simulink model "ActvnCoorrForEcuAndCom"  (R2022b)
settings: Solver=FixedStepDiscrete  StartTime=0.0  StopTime=10.0  FixedStep=0.1  RelTol=1e-3
settings Model: Creator=blue.ge  LastModifiedBy=blue.ge
settings DataIOCC: SaveFormat=StructureWithTime
settings OptimizationCC: DefaultParameterBehavior=Inlined  EnhancedBackFolding=on  PassReuseOutputArgsAs=Individual arguments  ZeroExternalMemoryAtStartup=off  ZeroInternalMemoryAtStartup=off  OptimizeBlockOrder=speed  DifferentSizesBufferReuse=on  OptimizationCustomize=off  EfficientTunableParamExpr=on
settings DebuggingCC: ParameterTunabilityLossMsg=error
settings ModelReferenceCC: ModelReferenceNumInstancesAllowed=Single
settings SFSimCC: MATLABDynamicMemAlloc=off
settings RTWCC: Description=AUTOSAR  SystemTargetFile=autosar.tlc  GenCodeOnly=on  TemplateMakefile=ert_default_tmf
settings CodeAppCC: ForceParamTrailComments=on  IgnoreCustomStorageClasses=off  OperatorAnnotations=on  SimulinkDataObjDesc=on  SFDataObjDesc=on  InsertBlockDesc=on
settings STFCustomTargetCC: TargetLangStandard=C99 (ISO)  MultiwordLength=256  ParMdlRefBuildCompliant=on  CompOptLevelCompliant=on  IncludeMdlTerminateFcn=off  CombineOutputUpdateFcns=on  SuppressErrorStatus=on  ERTCustomFileBanners=on  SupportNonFinite=off  SupportComplex=off  SupportContinuousTime=off  SupportNonInlinedSFcns=off  RemoveResetFunc=on  MATLABClassNameForMDSCustomization=Simulink.SoftwareTarget.ERTCustomization  AutosarCompliant=on  UseToolchainInfoCompliant=on  LookupTableObjectStructAxisOrder=2,1,3,4,...  LUTObjectStructOrderEvenSpacing=Size,Table,Breakpoints  SystemTargetFile=autosar.tlc
settings ERTTargetCC: TargetLangStandard=C99 (ISO)  ConcurrentExecutionCompliant=off  IncludeMdlTerminateFcn=off  SuppressErrorStatus=on  SupportNonFinite=off  SupportComplex=off  ModelStepFunctionPrototypeControlCompliant=off  CPPClassGenCompliant=off  AutosarCompliant=on  LookupTableObjectStructAxisOrder=2,1,3,4,...  LUTObjectStructOrderEvenSpacing=Size,Table,Breakpoints  InlinedParameterPlacement=NonHierarchical  ERTCustomFileTemplate=example_file_process.tlc

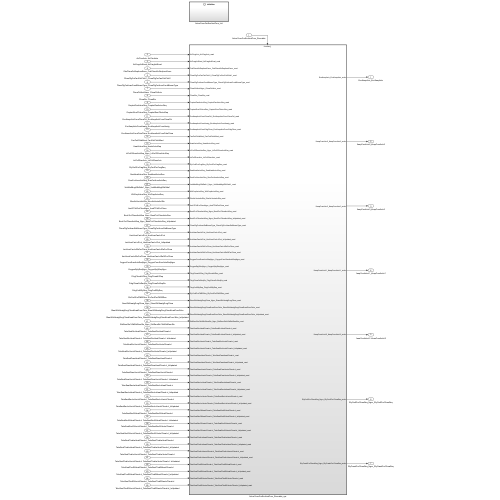
[thumbnail: Simulink's stored preview of the top-level diagram]
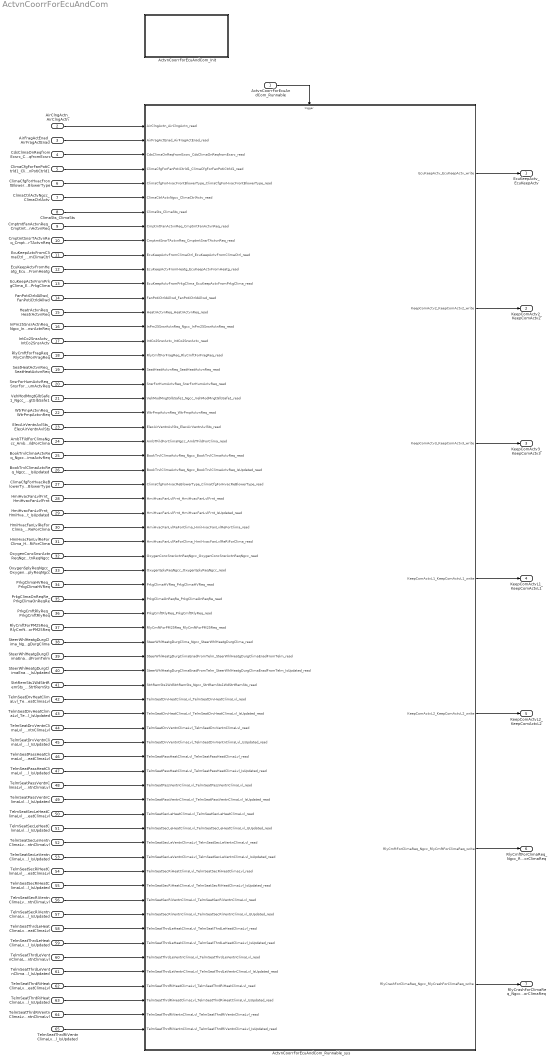
== system ActvnCoorrForEcuAndCom ==
  ActvnCoorrForEcuAndCom_Runnable : Inport  SampleTime=0.1  OutputFunctionCall=on
  AirClngActn_AirClngActn : Inport  Port=2  OutDataTypeStr=Enum: OnOff1  PortDimensions=1
  AirFragActEnad_AirFragActEnad : Inport  Port=3  OutDataTypeStr=Enum: OnOff1  PortDimensions=1
  CdsClimaOnReqfromExsrc_CdsClimaOnReqfromExsrc : Inport  Port=4  OutDataTypeStr=Enum: OnOff1  PortDimensions=1
  ClimaCfgForFanPotiCtrld1_ClimaCfgForFanPotiCtrld1 : Inport  Port=5  OutDataTypeStr=Boolean  PortDimensions=1
  ClimaCfgForHvacFrontBlowerType_ClimaCfgForHvacFrontBlowerType : Inport  Port=6  OutDataTypeStr=Enum: ClimaCfgForHvacFrontBlowerType  PortDimensions=1
  ClimaCtrlActvNgcc_ClimaCtrlActv : Inport  Port=7  OutDataTypeStr=Boolean  PortDimensions=1
  ClimaSts_ClimaSts : Inport  Port=8  OutDataTypeStr=Enum: ClimaSts  PortDimensions=1
  CmptmtFanActvnReq_CmptmtFanActvnReq : Inport  Port=9  OutDataTypeStr=Enum: OnOff1  PortDimensions=1
  CmptmtSnsrTActvnReq_CmptmtSnsrTActvnReq : Inport  Port=10  OutDataTypeStr=Enum: OnOff1  PortDimensions=1
  EcuKeepActvFromClimaCtrl_EcuKeepActvFromClimaCtrl : Inport  Port=11  OutDataTypeStr=Enum: OnOff1  PortDimensions=1
  EcuKeepActvFromHeatg_EcuKeepActvFromHeatg : Inport  Port=12  OutDataTypeStr=Enum: OnOff1  PortDimensions=1
  EcuKeepActvFromPrkgClima_EcuKeepActvFromPrkgClima : Inport  Port=13  OutDataTypeStr=Boolean  PortDimensions=1
  FanPotiCtrldAllwd_FanPotiCtrldAllwd : Inport  Port=14  OutDataTypeStr=Boolean  PortDimensions=1
  HeatrActvnReq_HeatrActvnReq : Inport  Port=15  OutDataTypeStr=Enum: OnOff1  PortDimensions=1
  InPm25SnsrActnReq_Ngcc_InPm25SnsrActnReq : Inport  Port=16  OutDataTypeStr=Enum: OnOff1  PortDimensions=1
  IntCo2SnsrActv_IntCo2SnsrActv : Inport  Port=17  OutDataTypeStr=Enum: ReqSts1  PortDimensions=1
  RlyCmftForFragReq_RlyCmftForFragReq : Inport  Port=18  OutDataTypeStr=Enum: OnOff1  PortDimensions=1
  SeatHeatActvnReq_SeatHeatActvnReq : Inport  Port=19  OutDataTypeStr=Enum: OnOff1  PortDimensions=1
  SnsrForHumActvReq_SnsrForHumActvReq : Inport  Port=20  OutDataTypeStr=Enum: OnOff1  PortDimensions=1
  VehModMngtGlbSafe1_Ngcc_VehModMngtGlbSafe1 : Inport  Port=21  OutDataTypeStr=Bus: VehModMngtGlbSafe1  PortDimensions=1  BusOutputAsStruct=on
  WtrPmpActvnReq_WtrPmpActvnReq : Inport  Port=22  OutDataTypeStr=Enum: OnOff1  PortDimensions=1
  ElecAirVentnAvlSts_ElecAirVentnAvlSts : Inport  Port=23  OutDataTypeStr=Enum: StsFd  PortDimensions=1
  AmbTFildForClimaNgcc_AmbTFildForClima : Inport  Port=24  OutDataTypeStr=T30  PortDimensions=1
  BookTrvlClimaActvReq_Ngcc_BookTrvlClimaActvReq : Inport  Port=25  OutDataTypeStr=Enum: OnOffNoReq  PortDimensions=1
  BookTrvlClimaActvReq_Ngcc_BookTrvlClimaActvReq_IsUpdated : Inport  Port=26  OutDataTypeStr=boolean  PortDimensions=1
  ClimaCfgForHvacReBlowerType_ClimaCfgForHvacReBlowerType : Inport  Port=27  OutDataTypeStr=Enum: ClimaCfgForHvacReBlowerType  PortDimensions=1
  HmiHvacFanLvlFrnt_HmiHvacFanLvlFrnt : Inport  Port=28  OutDataTypeStr=Enum: HmiHvacFanLvl  PortDimensions=1
  HmiHvacFanLvlFrnt_HmiHvacFanLvlFrnt_IsUpdated : Inport  Port=29  OutDataTypeStr=boolean  PortDimensions=1
  HmiHvacFanLvlReForClima_HmiHvacFanLvlReForClima : Inport  Port=30  OutDataTypeStr=Enum: HmiHvacFanLvl  PortDimensions=1
  HmiHvacFanLvlReForClima_HmiHvacFanLvlReRiForClima : Inport  Port=31  OutDataTypeStr=Enum: HmiHvacFanLvl  PortDimensions=1
  OxygenConcSnsrActnReqNgcc_OxygenConcSnsrActnReqNgcc : Inport  Port=32  OutDataTypeStr=Enum: OnOff1  PortDimensions=1
  OxygenSplyReqNgcc_OxygenSplyReqNgcc : Inport  Port=33  OutDataTypeStr=Enum: OxygenSplyReq  PortDimensions=1
  PrkgClimaHVReq_PrkgClimaHVReq : Inport  Port=34  OutDataTypeStr=Enum: OnOff1  PortDimensions=1
  PrkgClimaOnReqRe_PrkgClimaOnReqRe : Inport  Port=35  OutDataTypeStr=Enum: OnOff1  PortDimensions=1
  PrkgCmftRlyReq_PrkgCmftRlyReq : Inport  Port=36  OutDataTypeStr=Boolean  PortDimensions=1
  RlyCmftForPM25Req_RlyCmftForPM25Req : Inport  Port=37  OutDataTypeStr=Enum: OnOff1  PortDimensions=1
  SteerWhlHeatgDurgClima_Ngcc_SteerWhlHeatgDurgClima : Inport  Port=38  OutDataTypeStr=Boolean  PortDimensions=1
  SteerWhlHeatgDurgClimaEnadFromTelm_SteerWhlHeatgDurgClimaEnadFromTelm : Inport  Port=39  OutDataTypeStr=Enum: OnOff1  PortDimensions=1
  SteerWhlHeatgDurgClimaEnadFromTelm_SteerWhlHeatgDurgClimaEnadFromTelm_IsUpdated : Inport  Port=40  OutDataTypeStr=boolean  PortDimensions=1
  StrtRemSts1WdStrtRemSts_Ngcc_StrtRemSts1WdStrtRemSts : Inport  Port=41  OutDataTypeStr=Enum: StrtRemSts1  PortDimensions=1
  TelmSeatDrvHeatClimaLvl_TelmSeatDrvHeatClimaLvl : Inport  Port=42  OutDataTypeStr=Enum: TelmSeatClimaLvl  PortDimensions=1
  TelmSeatDrvHeatClimaLvl_TelmSeatDrvHeatClimaLvl_IsUpdated : Inport  Port=43  OutDataTypeStr=boolean  PortDimensions=1
  TelmSeatDrvVentnClimaLvl_TelmSeatDrvVentnClimaLvl : Inport  Port=44  OutDataTypeStr=Enum: TelmSeatClimaLvl  PortDimensions=1
  TelmSeatDrvVentnClimaLvl_TelmSeatDrvVentnClimaLvl_IsUpdated : Inport  Port=45  OutDataTypeStr=boolean  PortDimensions=1
  TelmSeatPassHeatClimaLvl_TelmSeatPassHeatClimaLvl : Inport  Port=46  OutDataTypeStr=Enum: TelmSeatClimaLvl  PortDimensions=1
  TelmSeatPassHeatClimaLvl_TelmSeatPassHeatClimaLvl_IsUpdated : Inport  Port=47  OutDataTypeStr=boolean  PortDimensions=1
  TelmSeatPassVentnClimaLvl_TelmSeatPassVentnClimaLvl : Inport  Port=48  OutDataTypeStr=Enum: TelmSeatClimaLvl  PortDimensions=1
  TelmSeatPassVentnClimaLvl_TelmSeatPassVentnClimaLvl_IsUpdated : Inport  Port=49  OutDataTypeStr=boolean  PortDimensions=1
  TelmSeatSecLeHeatClimaLvl_TelmSeatSecLeHeatClimaLvl : Inport  Port=50  OutDataTypeStr=Enum: TelmSeatClimaLvl  PortDimensions=1
  TelmSeatSecLeHeatClimaLvl_TelmSeatSecLeHeatClimaLvl_IsUpdated : Inport  Port=51  OutDataTypeStr=boolean  PortDimensions=1
  TelmSeatSecLeVentnClimaLvl_TelmSeatSecLeVentnClimaLvl : Inport  Port=52  OutDataTypeStr=Enum: TelmSeatClimaLvl  PortDimensions=1
  TelmSeatSecLeVentnClimaLvl_TelmSeatSecLeVentnClimaLvl_IsUpdated : Inport  Port=53  OutDataTypeStr=boolean  PortDimensions=1
  TelmSeatSecRiHeatClimaLvl_TelmSeatSecRiHeatClimaLvl : Inport  Port=54  OutDataTypeStr=Enum: TelmSeatClimaLvl  PortDimensions=1
  TelmSeatSecRiHeatClimaLvl_TelmSeatSecRiHeatClimaLvl_IsUpdated : Inport  Port=55  OutDataTypeStr=boolean  PortDimensions=1
  TelmSeatSecRiVentnClimaLvl_TelmSeatSecRiVentnClimaLvl : Inport  Port=56  OutDataTypeStr=Enum: TelmSeatClimaLvl  PortDimensions=1
  TelmSeatSecRiVentnClimaLvl_TelmSeatSecRiVentnClimaLvl_IsUpdated : Inport  Port=57  OutDataTypeStr=boolean  PortDimensions=1
  TelmSeatThrdLeHeatClimaLvl_TelmSeatThrdLeHeatClimaLvl : Inport  Port=58  OutDataTypeStr=Enum: TelmSeatClimaLvl  PortDimensions=1
  TelmSeatThrdLeHeatClimaLvl_TelmSeatThrdLeHeatClimaLvl_IsUpdated : Inport  Port=59  OutDataTypeStr=boolean  PortDimensions=1
  TelmSeatThrdLeVentnClimaLvl_TelmSeatThrdLeVentnClimaLvl : Inport  Port=60  OutDataTypeStr=Enum: TelmSeatClimaLvl  PortDimensions=1
  TelmSeatThrdLeVentnClimaLvl_TelmSeatThrdLeVentnClimaLvl_IsUpdated : Inport  Port=61  OutDataTypeStr=boolean  PortDimensions=1
  TelmSeatThrdRiHeatClimaLvl_TelmSeatThrdRiHeatClimaLvl : Inport  Port=62  OutDataTypeStr=Enum: TelmSeatClimaLvl  PortDimensions=1
  TelmSeatThrdRiHeatClimaLvl_TelmSeatThrdRiHeatClimaLvl_IsUpdated : Inport  Port=63  OutDataTypeStr=boolean  PortDimensions=1
  TelmSeatThrdRiVentnClimaLvl_TelmSeatThrdRiVentnClimaLvl : Inport  Port=64  OutDataTypeStr=Enum: TelmSeatClimaLvl  PortDimensions=1
  TelmSeatThrdRiVentnClimaLvl_TelmSeatThrdRiVentnClimaLvl_IsUpdated : Inport  Port=65  OutDataTypeStr=boolean  PortDimensions=1
  ActvnCoorrForEcuAndCom_Init : Subsystem
  ActvnCoorrForEcuAndCom_Runnable_sys : Subsystem
  EcuKeepActv_EcuKeepActv : Outport  OutDataTypeStr=Enum: OnOff1  PortDimensions=1
  KeepComActv2_KeepComActv2 : Outport  Port=2  OutDataTypeStr=Enum: OnOff1  PortDimensions=1
  KeepComActv3_KeepComActv3 : Outport  Port=3  OutDataTypeStr=Enum: OnOff1  PortDimensions=1
  KeepComActvL1_KeepComActvL1 : Outport  Port=4  OutDataTypeStr=Enum: OnOff1  PortDimensions=1
  KeepComActvL2_KeepComActvL2 : Outport  Port=5  OutDataTypeStr=Enum: OnOff1  PortDimensions=1
  RlyCmftForClimaReq_Ngcc_RlyCmftForClimaReq : Outport  Port=6  OutDataTypeStr=Enum: OnOff1  PortDimensions=1
  RlyCrashForClimaReq_Ngcc_RlyCrashForClimaReq : Outport  Port=7  OutDataTypeStr=Enum: OnOff1  PortDimensions=1
  wires:
  AirClngActn_AirClngActn/1 -> ActvnCoorrForEcuAndCom_Runnable_sys/1(AirClngActn_AirClngActn_read)
  AirFragActEnad_AirFragActEnad/1 -> ActvnCoorrForEcuAndCom_Runnable_sys/2(AirFragActEnad_AirFragActEnad_read)
  CdsClimaOnReqfromExsrc_CdsClimaOnReqfromExsrc/1 -> ActvnCoorrForEcuAndCom_Runnable_sys/3(CdsClimaOnReqfromExsrc_CdsClimaOnReqfromExsrc_read)
  ClimaCfgForFanPotiCtrld1_ClimaCfgForFanPotiCtrld1/1 -> ActvnCoorrForEcuAndCom_Runnable_sys/4(ClimaCfgForFanPotiCtrld1_ClimaCfgForFanPotiCtrld1_read)
  ClimaCfgForHvacFrontBlowerType_ClimaCfgForHvacFrontBlowerType/1 -> ActvnCoorrForEcuAndCom_Runnable_sys/5(ClimaCfgForHvacFrontBlowerType_ClimaCfgForHvacFrontBlowerType_read)
  ClimaCtrlActvNgcc_ClimaCtrlActv/1 -> ActvnCoorrForEcuAndCom_Runnable_sys/6(ClimaCtrlActvNgcc_ClimaCtrlActv_read)
  ClimaSts_ClimaSts/1 -> ActvnCoorrForEcuAndCom_Runnable_sys/7(ClimaSts_ClimaSts_read)
  CmptmtFanActvnReq_CmptmtFanActvnReq/1 -> ActvnCoorrForEcuAndCom_Runnable_sys/8(CmptmtFanActvnReq_CmptmtFanActvnReq_read)
  CmptmtSnsrTActvnReq_CmptmtSnsrTActvnReq/1 -> ActvnCoorrForEcuAndCom_Runnable_sys/9(CmptmtSnsrTActvnReq_CmptmtSnsrTActvnReq_read)
  EcuKeepActvFromClimaCtrl_EcuKeepActvFromClimaCtrl/1 -> ActvnCoorrForEcuAndCom_Runnable_sys/10(EcuKeepActvFromClimaCtrl_EcuKeepActvFromClimaCtrl_read)
  EcuKeepActvFromHeatg_EcuKeepActvFromHeatg/1 -> ActvnCoorrForEcuAndCom_Runnable_sys/11(EcuKeepActvFromHeatg_EcuKeepActvFromHeatg_read)
  EcuKeepActvFromPrkgClima_EcuKeepActvFromPrkgClima/1 -> ActvnCoorrForEcuAndCom_Runnable_sys/12(EcuKeepActvFromPrkgClima_EcuKeepActvFromPrkgClima_read)
  FanPotiCtrldAllwd_FanPotiCtrldAllwd/1 -> ActvnCoorrForEcuAndCom_Runnable_sys/13(FanPotiCtrldAllwd_FanPotiCtrldAllwd_read)
  HeatrActvnReq_HeatrActvnReq/1 -> ActvnCoorrForEcuAndCom_Runnable_sys/14(HeatrActvnReq_HeatrActvnReq_read)
  InPm25SnsrActnReq_Ngcc_InPm25SnsrActnReq/1 -> ActvnCoorrForEcuAndCom_Runnable_sys/15(InPm25SnsrActnReq_Ngcc_InPm25SnsrActnReq_read)
  IntCo2SnsrActv_IntCo2SnsrActv/1 -> ActvnCoorrForEcuAndCom_Runnable_sys/16(IntCo2SnsrActv_IntCo2SnsrActv_read)
  RlyCmftForFragReq_RlyCmftForFragReq/1 -> ActvnCoorrForEcuAndCom_Runnable_sys/17(RlyCmftForFragReq_RlyCmftForFragReq_read)
  SeatHeatActvnReq_SeatHeatActvnReq/1 -> ActvnCoorrForEcuAndCom_Runnable_sys/18(SeatHeatActvnReq_SeatHeatActvnReq_read)
  SnsrForHumActvReq_SnsrForHumActvReq/1 -> ActvnCoorrForEcuAndCom_Runnable_sys/19(SnsrForHumActvReq_SnsrForHumActvReq_read)
  VehModMngtGlbSafe1_Ngcc_VehModMngtGlbSafe1/1 -> ActvnCoorrForEcuAndCom_Runnable_sys/20(VehModMngtGlbSafe1_Ngcc_VehModMngtGlbSafe1_read)
  WtrPmpActvnReq_WtrPmpActvnReq/1 -> ActvnCoorrForEcuAndCom_Runnable_sys/21(WtrPmpActvnReq_WtrPmpActvnReq_read)
  ElecAirVentnAvlSts_ElecAirVentnAvlSts/1 -> ActvnCoorrForEcuAndCom_Runnable_sys/22(ElecAirVentnAvlSts_ElecAirVentnAvlSts_read)
  AmbTFildForClimaNgcc_AmbTFildForClima/1 -> ActvnCoorrForEcuAndCom_Runnable_sys/23(AmbTFildForClimaNgcc_AmbTFildForClima_read)
  BookTrvlClimaActvReq_Ngcc_BookTrvlClimaActvReq/1 -> ActvnCoorrForEcuAndCom_Runnable_sys/24(BookTrvlClimaActvReq_Ngcc_BookTrvlClimaActvReq_read)
  BookTrvlClimaActvReq_Ngcc_BookTrvlClimaActvReq_IsUpdated/1 -> ActvnCoorrForEcuAndCom_Runnable_sys/25(BookTrvlClimaActvReq_Ngcc_BookTrvlClimaActvReq_IsUpdated_read)
  ClimaCfgForHvacReBlowerType_ClimaCfgForHvacReBlowerType/1 -> ActvnCoorrForEcuAndCom_Runnable_sys/26(ClimaCfgForHvacReBlowerType_ClimaCfgForHvacReBlowerType_read)
  HmiHvacFanLvlFrnt_HmiHvacFanLvlFrnt/1 -> ActvnCoorrForEcuAndCom_Runnable_sys/27(HmiHvacFanLvlFrnt_HmiHvacFanLvlFrnt_read)
  HmiHvacFanLvlFrnt_HmiHvacFanLvlFrnt_IsUpdated/1 -> ActvnCoorrForEcuAndCom_Runnable_sys/28(HmiHvacFanLvlFrnt_HmiHvacFanLvlFrnt_IsUpdated_read)
  HmiHvacFanLvlReForClima_HmiHvacFanLvlReForClima/1 -> ActvnCoorrForEcuAndCom_Runnable_sys/29(HmiHvacFanLvlReForClima_HmiHvacFanLvlReForClima_read)
  HmiHvacFanLvlReForClima_HmiHvacFanLvlReRiForClima/1 -> ActvnCoorrForEcuAndCom_Runnable_sys/30(HmiHvacFanLvlReForClima_HmiHvacFanLvlReRiForClima_read)
  OxygenConcSnsrActnReqNgcc_OxygenConcSnsrActnReqNgcc/1 -> ActvnCoorrForEcuAndCom_Runnable_sys/31(OxygenConcSnsrActnReqNgcc_OxygenConcSnsrActnReqNgcc_read)
  OxygenSplyReqNgcc_OxygenSplyReqNgcc/1 -> ActvnCoorrForEcuAndCom_Runnable_sys/32(OxygenSplyReqNgcc_OxygenSplyReqNgcc_read)
  PrkgClimaHVReq_PrkgClimaHVReq/1 -> ActvnCoorrForEcuAndCom_Runnable_sys/33(PrkgClimaHVReq_PrkgClimaHVReq_read)
  PrkgClimaOnReqRe_PrkgClimaOnReqRe/1 -> ActvnCoorrForEcuAndCom_Runnable_sys/34(PrkgClimaOnReqRe_PrkgClimaOnReqRe_read)
  PrkgCmftRlyReq_PrkgCmftRlyReq/1 -> ActvnCoorrForEcuAndCom_Runnable_sys/35(PrkgCmftRlyReq_PrkgCmftRlyReq_read)
  RlyCmftForPM25Req_RlyCmftForPM25Req/1 -> ActvnCoorrForEcuAndCom_Runnable_sys/36(RlyCmftForPM25Req_RlyCmftForPM25Req_read)
  SteerWhlHeatgDurgClima_Ngcc_SteerWhlHeatgDurgClima/1 -> ActvnCoorrForEcuAndCom_Runnable_sys/37(SteerWhlHeatgDurgClima_Ngcc_SteerWhlHeatgDurgClima_read)
  SteerWhlHeatgDurgClimaEnadFromTelm_SteerWhlHeatgDurgClimaEnadFromTelm/1 -> ActvnCoorrForEcuAndCom_Runnable_sys/38(SteerWhlHeatgDurgClimaEnadFromTelm_SteerWhlHeatgDurgClimaEnadFromTelm_read)
  SteerWhlHeatgDurgClimaEnadFromTelm_SteerWhlHeatgDurgClimaEnadFromTelm_IsUpdated/1 -> ActvnCoorrForEcuAndCom_Runnable_sys/39(SteerWhlHeatgDurgClimaEnadFromTelm_SteerWhlHeatgDurgClimaEnadFromTelm_IsUpdated_read)
  StrtRemSts1WdStrtRemSts_Ngcc_StrtRemSts1WdStrtRemSts/1 -> ActvnCoorrForEcuAndCom_Runnable_sys/40(StrtRemSts1WdStrtRemSts_Ngcc_StrtRemSts1WdStrtRemSts_read)
  TelmSeatDrvHeatClimaLvl_TelmSeatDrvHeatClimaLvl/1 -> ActvnCoorrForEcuAndCom_Runnable_sys/41(TelmSeatDrvHeatClimaLvl_TelmSeatDrvHeatClimaLvl_read)
  TelmSeatDrvHeatClimaLvl_TelmSeatDrvHeatClimaLvl_IsUpdated/1 -> ActvnCoorrForEcuAndCom_Runnable_sys/42(TelmSeatDrvHeatClimaLvl_TelmSeatDrvHeatClimaLvl_IsUpdated_read)
  TelmSeatDrvVentnClimaLvl_TelmSeatDrvVentnClimaLvl/1 -> ActvnCoorrForEcuAndCom_Runnable_sys/43(TelmSeatDrvVentnClimaLvl_TelmSeatDrvVentnClimaLvl_read)
  TelmSeatDrvVentnClimaLvl_TelmSeatDrvVentnClimaLvl_IsUpdated/1 -> ActvnCoorrForEcuAndCom_Runnable_sys/44(TelmSeatDrvVentnClimaLvl_TelmSeatDrvVentnClimaLvl_IsUpdated_read)
  TelmSeatPassHeatClimaLvl_TelmSeatPassHeatClimaLvl/1 -> ActvnCoorrForEcuAndCom_Runnable_sys/45(TelmSeatPassHeatClimaLvl_TelmSeatPassHeatClimaLvl_read)
  TelmSeatPassHeatClimaLvl_TelmSeatPassHeatClimaLvl_IsUpdated/1 -> ActvnCoorrForEcuAndCom_Runnable_sys/46(TelmSeatPassHeatClimaLvl_TelmSeatPassHeatClimaLvl_IsUpdated_read)
  TelmSeatPassVentnClimaLvl_TelmSeatPassVentnClimaLvl/1 -> ActvnCoorrForEcuAndCom_Runnable_sys/47(TelmSeatPassVentnClimaLvl_TelmSeatPassVentnClimaLvl_read)
  TelmSeatPassVentnClimaLvl_TelmSeatPassVentnClimaLvl_IsUpdated/1 -> ActvnCoorrForEcuAndCom_Runnable_sys/48(TelmSeatPassVentnClimaLvl_TelmSeatPassVentnClimaLvl_IsUpdated_read)
  TelmSeatSecLeHeatClimaLvl_TelmSeatSecLeHeatClimaLvl/1 -> ActvnCoorrForEcuAndCom_Runnable_sys/49(TelmSeatSecLeHeatClimaLvl_TelmSeatSecLeHeatClimaLvl_read)
  TelmSeatSecLeHeatClimaLvl_TelmSeatSecLeHeatClimaLvl_IsUpdated/1 -> ActvnCoorrForEcuAndCom_Runnable_sys/50(TelmSeatSecLeHeatClimaLvl_TelmSeatSecLeHeatClimaLvl_IsUpdated_read)
  TelmSeatSecLeVentnClimaLvl_TelmSeatSecLeVentnClimaLvl/1 -> ActvnCoorrForEcuAndCom_Runnable_sys/51(TelmSeatSecLeVentnClimaLvl_TelmSeatSecLeVentnClimaLvl_read)
  TelmSeatSecLeVentnClimaLvl_TelmSeatSecLeVentnClimaLvl_IsUpdated/1 -> ActvnCoorrForEcuAndCom_Runnable_sys/52(TelmSeatSecLeVentnClimaLvl_TelmSeatSecLeVentnClimaLvl_IsUpdated_read)
  TelmSeatSecRiHeatClimaLvl_TelmSeatSecRiHeatClimaLvl/1 -> ActvnCoorrForEcuAndCom_Runnable_sys/53(TelmSeatSecRiHeatClimaLvl_TelmSeatSecRiHeatClimaLvl_read)
  TelmSeatSecRiHeatClimaLvl_TelmSeatSecRiHeatClimaLvl_IsUpdated/1 -> ActvnCoorrForEcuAndCom_Runnable_sys/54(TelmSeatSecRiHeatClimaLvl_TelmSeatSecRiHeatClimaLvl_IsUpdated_read)
  TelmSeatSecRiVentnClimaLvl_TelmSeatSecRiVentnClimaLvl/1 -> ActvnCoorrForEcuAndCom_Runnable_sys/55(TelmSeatSecRiVentnClimaLvl_TelmSeatSecRiVentnClimaLvl_read)
  TelmSeatSecRiVentnClimaLvl_TelmSeatSecRiVentnClimaLvl_IsUpdated/1 -> ActvnCoorrForEcuAndCom_Runnable_sys/56(TelmSeatSecRiVentnClimaLvl_TelmSeatSecRiVentnClimaLvl_IsUpdated_read)
  TelmSeatThrdLeHeatClimaLvl_TelmSeatThrdLeHeatClimaLvl/1 -> ActvnCoorrForEcuAndCom_Runnable_sys/57(TelmSeatThrdLeHeatClimaLvl_TelmSeatThrdLeHeatClimaLvl_read)
  TelmSeatThrdLeHeatClimaLvl_TelmSeatThrdLeHeatClimaLvl_IsUpdated/1 -> ActvnCoorrForEcuAndCom_Runnable_sys/58(TelmSeatThrdLeHeatClimaLvl_TelmSeatThrdLeHeatClimaLvl_IsUpdated_read)
  TelmSeatThrdLeVentnClimaLvl_TelmSeatThrdLeVentnClimaLvl/1 -> ActvnCoorrForEcuAndCom_Runnable_sys/59(TelmSeatThrdLeVentnClimaLvl_TelmSeatThrdLeVentnClimaLvl_read)
  TelmSeatThrdLeVentnClimaLvl_TelmSeatThrdLeVentnClimaLvl_IsUpdated/1 -> ActvnCoorrForEcuAndCom_Runnable_sys/60(TelmSeatThrdLeVentnClimaLvl_TelmSeatThrdLeVentnClimaLvl_IsUpdated_read)
  TelmSeatThrdRiHeatClimaLvl_TelmSeatThrdRiHeatClimaLvl/1 -> ActvnCoorrForEcuAndCom_Runnable_sys/61(TelmSeatThrdRiHeatClimaLvl_TelmSeatThrdRiHeatClimaLvl_read)
  TelmSeatThrdRiHeatClimaLvl_TelmSeatThrdRiHeatClimaLvl_IsUpdated/1 -> ActvnCoorrForEcuAndCom_Runnable_sys/62(TelmSeatThrdRiHeatClimaLvl_TelmSeatThrdRiHeatClimaLvl_IsUpdated_read)
  TelmSeatThrdRiVentnClimaLvl_TelmSeatThrdRiVentnClimaLvl/1 -> ActvnCoorrForEcuAndCom_Runnable_sys/63(TelmSeatThrdRiVentnClimaLvl_TelmSeatThrdRiVentnClimaLvl_read)
  TelmSeatThrdRiVentnClimaLvl_TelmSeatThrdRiVentnClimaLvl_IsUpdated/1 -> ActvnCoorrForEcuAndCom_Runnable_sys/64(TelmSeatThrdRiVentnClimaLvl_TelmSeatThrdRiVentnClimaLvl_IsUpdated_read)
  ActvnCoorrForEcuAndCom_Runnable_sys/1(EcuKeepActv_EcuKeepActv_write) -> EcuKeepActv_EcuKeepActv/1
  ActvnCoorrForEcuAndCom_Runnable_sys/2(KeepComActv2_KeepComActv2_write) -> KeepComActv2_KeepComActv2/1
  ActvnCoorrForEcuAndCom_Runnable_sys/3(KeepComActv3_KeepComActv3_write) -> KeepComActv3_KeepComActv3/1
  ActvnCoorrForEcuAndCom_Runnable_sys/4(KeepComActvL1_KeepComActvL1_write) -> KeepComActvL1_KeepComActvL1/1
  ActvnCoorrForEcuAndCom_Runnable_sys/5(KeepComActvL2_KeepComActvL2_write) -> KeepComActvL2_KeepComActvL2/1
  ActvnCoorrForEcuAndCom_Runnable_sys/6(RlyCmftForClimaReq_Ngcc_RlyCmftForClimaReq_write) -> RlyCmftForClimaReq_Ngcc_RlyCmftForClimaReq/1
  ActvnCoorrForEcuAndCom_Runnable_sys/7(RlyCrashForClimaReq_Ngcc_RlyCrashForClimaReq_write) -> RlyCrashForClimaReq_Ngcc_RlyCrashForClimaReq/1
  ActvnCoorrForEcuAndCom_Runnable/1 -> ActvnCoorrForEcuAndCom_Runnable_sys/Trigger

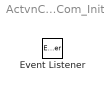
== system ActvnCoorrForEcuAndCom/ActvnCoorrForEcuAndCom_Init ==
  Event Listener : EventListener  EventName=reset

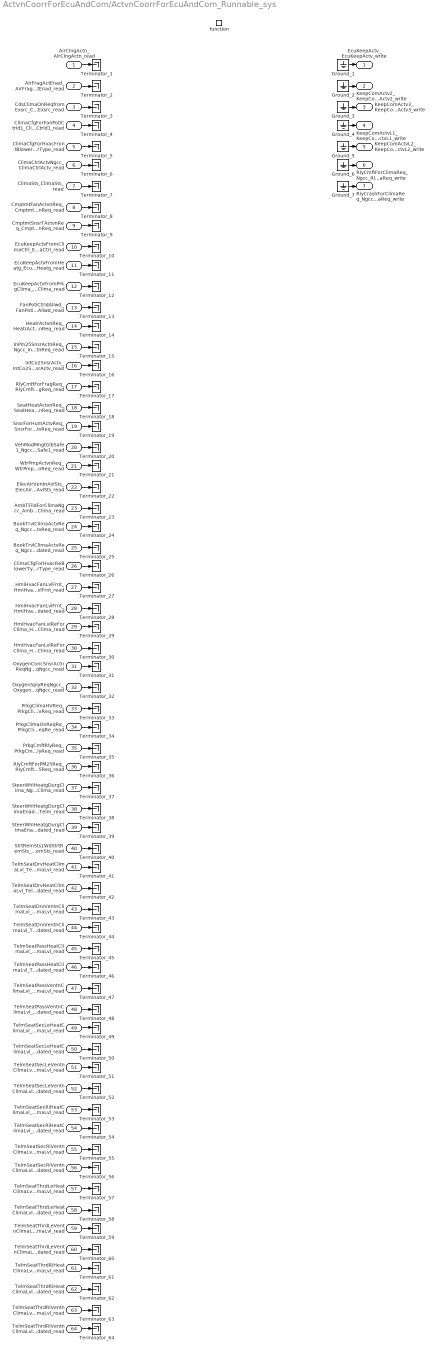
== system ActvnCoorrForEcuAndCom/ActvnCoorrForEcuAndCom_Runnable_sys ==
  AirClngActn_AirClngActn_read : Inport
  AirFragActEnad_AirFragActEnad_read : Inport  Port=2
  CdsClimaOnReqfromExsrc_CdsClimaOnReqfromExsrc_read : Inport  Port=3
  ClimaCfgForFanPotiCtrld1_ClimaCfgForFanPotiCtrld1_read : Inport  Port=4
  ClimaCfgForHvacFrontBlowerType_ClimaCfgForHvacFrontBlowerType_read : Inport  Port=5
  ClimaCtrlActvNgcc_ClimaCtrlActv_read : Inport  Port=6
  ClimaSts_ClimaSts_read : Inport  Port=7
  CmptmtFanActvnReq_CmptmtFanActvnReq_read : Inport  Port=8
  CmptmtSnsrTActvnReq_CmptmtSnsrTActvnReq_read : Inport  Port=9
  EcuKeepActvFromClimaCtrl_EcuKeepActvFromClimaCtrl_read : Inport  Port=10
  EcuKeepActvFromHeatg_EcuKeepActvFromHeatg_read : Inport  Port=11
  EcuKeepActvFromPrkgClima_EcuKeepActvFromPrkgClima_read : Inport  Port=12
  FanPotiCtrldAllwd_FanPotiCtrldAllwd_read : Inport  Port=13
  HeatrActvnReq_HeatrActvnReq_read : Inport  Port=14
  InPm25SnsrActnReq_Ngcc_InPm25SnsrActnReq_read : Inport  Port=15
  IntCo2SnsrActv_IntCo2SnsrActv_read : Inport  Port=16
  RlyCmftForFragReq_RlyCmftForFragReq_read : Inport  Port=17
  SeatHeatActvnReq_SeatHeatActvnReq_read : Inport  Port=18
  SnsrForHumActvReq_SnsrForHumActvReq_read : Inport  Port=19
  VehModMngtGlbSafe1_Ngcc_VehModMngtGlbSafe1_read : Inport  Port=20
  WtrPmpActvnReq_WtrPmpActvnReq_read : Inport  Port=21
  ElecAirVentnAvlSts_ElecAirVentnAvlSts_read : Inport  Port=22
  AmbTFildForClimaNgcc_AmbTFildForClima_read : Inport  Port=23
  BookTrvlClimaActvReq_Ngcc_BookTrvlClimaActvReq_read : Inport  Port=24
  BookTrvlClimaActvReq_Ngcc_BookTrvlClimaActvReq_IsUpdated_read : Inport  Port=25
  ClimaCfgForHvacReBlowerType_ClimaCfgForHvacReBlowerType_read : Inport  Port=26
  HmiHvacFanLvlFrnt_HmiHvacFanLvlFrnt_read : Inport  Port=27
  HmiHvacFanLvlFrnt_HmiHvacFanLvlFrnt_IsUpdated_read : Inport  Port=28
  HmiHvacFanLvlReForClima_HmiHvacFanLvlReForClima_read : Inport  Port=29
  HmiHvacFanLvlReForClima_HmiHvacFanLvlReRiForClima_read : Inport  Port=30
  OxygenConcSnsrActnReqNgcc_OxygenConcSnsrActnReqNgcc_read : Inport  Port=31
  OxygenSplyReqNgcc_OxygenSplyReqNgcc_read : Inport  Port=32
  PrkgClimaHVReq_PrkgClimaHVReq_read : Inport  Port=33
  PrkgClimaOnReqRe_PrkgClimaOnReqRe_read : Inport  Port=34
  PrkgCmftRlyReq_PrkgCmftRlyReq_read : Inport  Port=35
  RlyCmftForPM25Req_RlyCmftForPM25Req_read : Inport  Port=36
  SteerWhlHeatgDurgClima_Ngcc_SteerWhlHeatgDurgClima_read : Inport  Port=37
  SteerWhlHeatgDurgClimaEnadFromTelm_SteerWhlHeatgDurgClimaEnadFromTelm_read : Inport  Port=38
  SteerWhlHeatgDurgClimaEnadFromTelm_SteerWhlHeatgDurgClimaEnadFromTelm_IsUpdated_read : Inport  Port=39
  StrtRemSts1WdStrtRemSts_Ngcc_StrtRemSts1WdStrtRemSts_read : Inport  Port=40
  TelmSeatDrvHeatClimaLvl_TelmSeatDrvHeatClimaLvl_read : Inport  Port=41
  TelmSeatDrvHeatClimaLvl_TelmSeatDrvHeatClimaLvl_IsUpdated_read : Inport  Port=42
  TelmSeatDrvVentnClimaLvl_TelmSeatDrvVentnClimaLvl_read : Inport  Port=43
  TelmSeatDrvVentnClimaLvl_TelmSeatDrvVentnClimaLvl_IsUpdated_read : Inport  Port=44
  TelmSeatPassHeatClimaLvl_TelmSeatPassHeatClimaLvl_read : Inport  Port=45
  TelmSeatPassHeatClimaLvl_TelmSeatPassHeatClimaLvl_IsUpdated_read : Inport  Port=46
  TelmSeatPassVentnClimaLvl_TelmSeatPassVentnClimaLvl_read : Inport  Port=47
  TelmSeatPassVentnClimaLvl_TelmSeatPassVentnClimaLvl_IsUpdated_read : Inport  Port=48
  TelmSeatSecLeHeatClimaLvl_TelmSeatSecLeHeatClimaLvl_read : Inport  Port=49
  TelmSeatSecLeHeatClimaLvl_TelmSeatSecLeHeatClimaLvl_IsUpdated_read : Inport  Port=50
  TelmSeatSecLeVentnClimaLvl_TelmSeatSecLeVentnClimaLvl_read : Inport  Port=51
  TelmSeatSecLeVentnClimaLvl_TelmSeatSecLeVentnClimaLvl_IsUpdated_read : Inport  Port=52
  TelmSeatSecRiHeatClimaLvl_TelmSeatSecRiHeatClimaLvl_read : Inport  Port=53
  TelmSeatSecRiHeatClimaLvl_TelmSeatSecRiHeatClimaLvl_IsUpdated_read : Inport  Port=54
  TelmSeatSecRiVentnClimaLvl_TelmSeatSecRiVentnClimaLvl_read : Inport  Port=55
  TelmSeatSecRiVentnClimaLvl_TelmSeatSecRiVentnClimaLvl_IsUpdated_read : Inport  Port=56
  TelmSeatThrdLeHeatClimaLvl_TelmSeatThrdLeHeatClimaLvl_read : Inport  Port=57
  TelmSeatThrdLeHeatClimaLvl_TelmSeatThrdLeHeatClimaLvl_IsUpdated_read : Inport  Port=58
  TelmSeatThrdLeVentnClimaLvl_TelmSeatThrdLeVentnClimaLvl_read : Inport  Port=59
  TelmSeatThrdLeVentnClimaLvl_TelmSeatThrdLeVentnClimaLvl_IsUpdated_read : Inport  Port=60
  TelmSeatThrdRiHeatClimaLvl_TelmSeatThrdRiHeatClimaLvl_read : Inport  Port=61
  TelmSeatThrdRiHeatClimaLvl_TelmSeatThrdRiHeatClimaLvl_IsUpdated_read : Inport  Port=62
  TelmSeatThrdRiVentnClimaLvl_TelmSeatThrdRiVentnClimaLvl_read : Inport  Port=63
  TelmSeatThrdRiVentnClimaLvl_TelmSeatThrdRiVentnClimaLvl_IsUpdated_read : Inport  Port=64
  function : TriggerPort  TriggerType=function-call  VariantControl=(inherit)  SampleTimeType=periodic  SampleTime=-1
  Ground_{1..7} (7 alike) : Ground
  Terminator_{1..64} (64 alike) : Terminator
  EcuKeepActv_EcuKeepActv_write : Outport
      settings: EnsureOutportIsVirtual=on
  KeepComActv2_KeepComActv2_write : Outport  Port=2
      settings: EnsureOutportIsVirtual=on
  KeepComActv3_KeepComActv3_write : Outport  Port=3
      settings: EnsureOutportIsVirtual=on
  KeepComActvL1_KeepComActvL1_write : Outport  Port=4
      settings: EnsureOutportIsVirtual=on
  KeepComActvL2_KeepComActvL2_write : Outport  Port=5
      settings: EnsureOutportIsVirtual=on
  RlyCmftForClimaReq_Ngcc_RlyCmftForClimaReq_write : Outport  Port=6
      settings: EnsureOutportIsVirtual=on
  RlyCrashForClimaReq_Ngcc_RlyCrashForClimaReq_write : Outport  Port=7
      settings: EnsureOutportIsVirtual=on
  wires:
  AirClngActn_AirClngActn_read/1 -> Terminator_1/1
  AirFragActEnad_AirFragActEnad_read/1 -> Terminator_2/1
  CdsClimaOnReqfromExsrc_CdsClimaOnReqfromExsrc_read/1 -> Terminator_3/1
  ClimaCfgForFanPotiCtrld1_ClimaCfgForFanPotiCtrld1_read/1 -> Terminator_4/1
  ClimaCfgForHvacFrontBlowerType_ClimaCfgForHvacFrontBlowerType_read/1 -> Terminator_5/1
  ClimaCtrlActvNgcc_ClimaCtrlActv_read/1 -> Terminator_6/1
  ClimaSts_ClimaSts_read/1 -> Terminator_7/1
  CmptmtFanActvnReq_CmptmtFanActvnReq_read/1 -> Terminator_8/1
  CmptmtSnsrTActvnReq_CmptmtSnsrTActvnReq_read/1 -> Terminator_9/1
  EcuKeepActvFromClimaCtrl_EcuKeepActvFromClimaCtrl_read/1 -> Terminator_10/1
  EcuKeepActvFromHeatg_EcuKeepActvFromHeatg_read/1 -> Terminator_11/1
  EcuKeepActvFromPrkgClima_EcuKeepActvFromPrkgClima_read/1 -> Terminator_12/1
  FanPotiCtrldAllwd_FanPotiCtrldAllwd_read/1 -> Terminator_13/1
  HeatrActvnReq_HeatrActvnReq_read/1 -> Terminator_14/1
  InPm25SnsrActnReq_Ngcc_InPm25SnsrActnReq_read/1 -> Terminator_15/1
  IntCo2SnsrActv_IntCo2SnsrActv_read/1 -> Terminator_16/1
  RlyCmftForFragReq_RlyCmftForFragReq_read/1 -> Terminator_17/1
  SeatHeatActvnReq_SeatHeatActvnReq_read/1 -> Terminator_18/1
  SnsrForHumActvReq_SnsrForHumActvReq_read/1 -> Terminator_19/1
  VehModMngtGlbSafe1_Ngcc_VehModMngtGlbSafe1_read/1 -> Terminator_20/1
  WtrPmpActvnReq_WtrPmpActvnReq_read/1 -> Terminator_21/1
  ElecAirVentnAvlSts_ElecAirVentnAvlSts_read/1 -> Terminator_22/1
  AmbTFildForClimaNgcc_AmbTFildForClima_read/1 -> Terminator_23/1
  BookTrvlClimaActvReq_Ngcc_BookTrvlClimaActvReq_read/1 -> Terminator_24/1
  BookTrvlClimaActvReq_Ngcc_BookTrvlClimaActvReq_IsUpdated_read/1 -> Terminator_25/1
  ClimaCfgForHvacReBlowerType_ClimaCfgForHvacReBlowerType_read/1 -> Terminator_26/1
  HmiHvacFanLvlFrnt_HmiHvacFanLvlFrnt_read/1 -> Terminator_27/1
  HmiHvacFanLvlFrnt_HmiHvacFanLvlFrnt_IsUpdated_read/1 -> Terminator_28/1
  HmiHvacFanLvlReForClima_HmiHvacFanLvlReForClima_read/1 -> Terminator_29/1
  HmiHvacFanLvlReForClima_HmiHvacFanLvlReRiForClima_read/1 -> Terminator_30/1
  OxygenConcSnsrActnReqNgcc_OxygenConcSnsrActnReqNgcc_read/1 -> Terminator_31/1
  OxygenSplyReqNgcc_OxygenSplyReqNgcc_read/1 -> Terminator_32/1
  PrkgClimaHVReq_PrkgClimaHVReq_read/1 -> Terminator_33/1
  PrkgClimaOnReqRe_PrkgClimaOnReqRe_read/1 -> Terminator_34/1
  PrkgCmftRlyReq_PrkgCmftRlyReq_read/1 -> Terminator_35/1
  RlyCmftForPM25Req_RlyCmftForPM25Req_read/1 -> Terminator_36/1
  SteerWhlHeatgDurgClima_Ngcc_SteerWhlHeatgDurgClima_read/1 -> Terminator_37/1
  SteerWhlHeatgDurgClimaEnadFromTelm_SteerWhlHeatgDurgClimaEnadFromTelm_read/1 -> Terminator_38/1
  SteerWhlHeatgDurgClimaEnadFromTelm_SteerWhlHeatgDurgClimaEnadFromTelm_IsUpdated_read/1 -> Terminator_39/1
  StrtRemSts1WdStrtRemSts_Ngcc_StrtRemSts1WdStrtRemSts_read/1 -> Terminator_40/1
  TelmSeatDrvHeatClimaLvl_TelmSeatDrvHeatClimaLvl_read/1 -> Terminator_41/1
  TelmSeatDrvHeatClimaLvl_TelmSeatDrvHeatClimaLvl_IsUpdated_read/1 -> Terminator_42/1
  TelmSeatDrvVentnClimaLvl_TelmSeatDrvVentnClimaLvl_read/1 -> Terminator_43/1
  TelmSeatDrvVentnClimaLvl_TelmSeatDrvVentnClimaLvl_IsUpdated_read/1 -> Terminator_44/1
  TelmSeatPassHeatClimaLvl_TelmSeatPassHeatClimaLvl_read/1 -> Terminator_45/1
  TelmSeatPassHeatClimaLvl_TelmSeatPassHeatClimaLvl_IsUpdated_read/1 -> Terminator_46/1
  TelmSeatPassVentnClimaLvl_TelmSeatPassVentnClimaLvl_read/1 -> Terminator_47/1
  TelmSeatPassVentnClimaLvl_TelmSeatPassVentnClimaLvl_IsUpdated_read/1 -> Terminator_48/1
  TelmSeatSecLeHeatClimaLvl_TelmSeatSecLeHeatClimaLvl_read/1 -> Terminator_49/1
  TelmSeatSecLeHeatClimaLvl_TelmSeatSecLeHeatClimaLvl_IsUpdated_read/1 -> Terminator_50/1
  TelmSeatSecLeVentnClimaLvl_TelmSeatSecLeVentnClimaLvl_read/1 -> Terminator_51/1
  TelmSeatSecLeVentnClimaLvl_TelmSeatSecLeVentnClimaLvl_IsUpdated_read/1 -> Terminator_52/1
  TelmSeatSecRiHeatClimaLvl_TelmSeatSecRiHeatClimaLvl_read/1 -> Terminator_53/1
  TelmSeatSecRiHeatClimaLvl_TelmSeatSecRiHeatClimaLvl_IsUpdated_read/1 -> Terminator_54/1
  TelmSeatSecRiVentnClimaLvl_TelmSeatSecRiVentnClimaLvl_read/1 -> Terminator_55/1
  TelmSeatSecRiVentnClimaLvl_TelmSeatSecRiVentnClimaLvl_IsUpdated_read/1 -> Terminator_56/1
  TelmSeatThrdLeHeatClimaLvl_TelmSeatThrdLeHeatClimaLvl_read/1 -> Terminator_57/1
  TelmSeatThrdLeHeatClimaLvl_TelmSeatThrdLeHeatClimaLvl_IsUpdated_read/1 -> Terminator_58/1
  TelmSeatThrdLeVentnClimaLvl_TelmSeatThrdLeVentnClimaLvl_read/1 -> Terminator_59/1
  TelmSeatThrdLeVentnClimaLvl_TelmSeatThrdLeVentnClimaLvl_IsUpdated_read/1 -> Terminator_60/1
  TelmSeatThrdRiHeatClimaLvl_TelmSeatThrdRiHeatClimaLvl_read/1 -> Terminator_61/1
  TelmSeatThrdRiHeatClimaLvl_TelmSeatThrdRiHeatClimaLvl_IsUpdated_read/1 -> Terminator_62/1
  TelmSeatThrdRiVentnClimaLvl_TelmSeatThrdRiVentnClimaLvl_read/1 -> Terminator_63/1
  TelmSeatThrdRiVentnClimaLvl_TelmSeatThrdRiVentnClimaLvl_IsUpdated_read/1 -> Terminator_64/1
  Ground_1/1 -> EcuKeepActv_EcuKeepActv_write/1
  Ground_2/1 -> KeepComActv2_KeepComActv2_write/1
  Ground_3/1 -> KeepComActv3_KeepComActv3_write/1
  Ground_4/1 -> KeepComActvL1_KeepComActvL1_write/1
  Ground_5/1 -> KeepComActvL2_KeepComActvL2_write/1
  Ground_6/1 -> RlyCmftForClimaReq_Ngcc_RlyCmftForClimaReq_write/1
  Ground_7/1 -> RlyCrashForClimaReq_Ngcc_RlyCrashForClimaReq_write/1
